simulink model "CCMLoad"  (R2022b) (continued)
== system CCMLoad/CCMLoad_Runnable_sys == (continued)
  HvacCfgForClimaCtrl_HvacCfgForHvacReBlowerType_read : Inport  Port=50
  HvacCfgForClimaCtrl_HvacCfgForHvacReVentPstn_read : Inport  Port=51
  HvacCfgForClimaCtrl_HvacCfgForHvacSensorExistSts_read : Inport  Port=52
  HvacCfgForClimaCtrl_HvacCfgForHvacSnsrRCmptmtESts_read : Inport  Port=53
  HvacCfgForClimaCtrl_HvacCfgForHvacSnsrReHexESts_read : Inport  Port=54
  HvacFanDutyReqRe_HvacFanDutyReqRe_read : Inport  Port=55
  HvacFanReqFrnt_HvacFanReqFrnt_read : Inport  Port=56
  HvacFanSts2HvacFanMotU2_HvacFanSts2HvacFanMotU2_read : Inport  Port=57
  HvacFanVoltReqFrnt_HvacFanVoltReqFrnt_read : Inport  Port=58
  HvacFanVoltReqRe_HvacFanVoltReqRe_read : Inport  Port=59
  IDX_RETXVDiagStatus_read : Inport  Port=60
  ReEvapVlvReq_ReEvapVlvReq_read : Inport  Port=61
  ReVbmrSts_ReVbmrSts_read : Inport  Port=62
  RlyCmdCmft_RlyCmdCmft_read : Inport  Port=63
  RlyCmftForClimaReq_Ngcc_RlyCmftForClimaReq_read : Inport  Port=64
  RlyCrashForClimaReq_Ngcc_RlyCrashForClimaReq_read : Inport  Port=65
  SysServCarCfgSts_SysServCCPBGMNum_read : Inport  Port=66
  SysServCarCfgSts_SysServCCPLin2AQSSts_read : Inport  Port=67
  SysServCarCfgSts_SysServDUBlowerPwmSignTypeSts_read : Inport  Port=68
  SysServCarCfgSts_SysServDUDCFLMdeSts_read : Inport  Port=69
  SysServCarCfgSts_SysServDULin4HFAFStsH_read : Inport  Port=70
  SysServCarCfgSts_SysServDUReBlowerPwmSignSts_read : Inport  Port=71
  SysServCarCfgSts_SysServDUReBlowerPwmSts_read : Inport  Port=72
  SysServCarCfgSts_SysServFrntHEXSnsrPosn_read : Inport  Port=73
  SysServCarCfgSts_SysServLowSideRETXVSts_read : Inport  Port=74
  SysServCarCfgSts_SysServReHEXSnsrPosn_read : Inport  Port=75
  SysServCarCfgSts_SysServRHFASts_read : Inport  Port=76
  SysServCarCfgSts_SysServSnsrForFCmptmtTType_read : Inport  Port=77
  TiAndDateIndcn_TiAndDateIndcn_read : Inport  Port=78
  BswLifeCycle_LifeCycle_read : Inport  Port=79
  function : TriggerPort  TriggerType=function-call  VariantControl=(inherit)  SampleTimeType=periodic  SampleTime=-1
  AdServ_AdSampleValue : FunctionCaller  FunctionPrototype=[AdValue,ERR] = AdServ_AdSampleValue()  OutputArgumentSpecifications=P_SampleValueType, P_Std_ReturnType
  AdServ_AdSampleValueSts : FunctionCaller  FunctionPrototype=[AdValue,ERR] = AdServ_AdSampleValueSts()  OutputArgumentSpecifications=P_SampleValueTypeSts, P_Std_ReturnType
  AdServ_AdcSetup : FunctionCaller  FunctionPrototype=ERR = AdServ_AdcSetup()  OutputArgumentSpecifications=P_Std_ReturnType
  DioServ_PowerOffInitChannel : FunctionCaller  FunctionPrototype=ERR = DioServ_PowerOffInitChannel()  OutputArgumentSpecifications=P_Std_ReturnType
  DioServ_PowerOnInitChannel : FunctionCaller  FunctionPrototype=ERR = DioServ_PowerOnInitChannel()  OutputArgumentSpecifications=P_Std_ReturnType
  DioServ_ReadChannel : FunctionCaller  FunctionPrototype=[Level,ERR] = DioServ_ReadChannel(ChannelId)  InputArgumentSpecifications=DioChannelType(0)  OutputArgumentSpecifications=P_DioLevelType, P_Std_ReturnType
  DioServ_WriteChannel : FunctionCaller  FunctionPrototype=ERR = DioServ_WriteChannel(ChannelId,Level)  InputArgumentSpecifications=DioChannelType(0), P_DioLevelType  OutputArgumentSpecifications=P_Std_ReturnType
  Ground_{1..24} (24 alike) : Ground
  IcuServ_GetDutyCycleValues : FunctionCaller  FunctionPrototype=[DutyCycleValues,ERR] = IcuServ_GetDutyCycleValues(Channel)  InputArgumentSpecifications=IcuChannelType(0)  OutputArgumentSpecifications=P_IcuDutyCycleType, P_Std_ReturnType
  IcuServ_IcuStart : FunctionCaller  FunctionPrototype=ERR = IcuServ_IcuStart()  OutputArgumentSpecifications=P_Std_ReturnType
  IcuServ_SetMaxPwmPeriod : FunctionCaller  FunctionPrototype=ERR = IcuServ_SetMaxPwmPeriod(Channel,MaxPwmPeriod)  InputArgumentSpecifications=IcuChannelType(0), uint16(1)  OutputArgumentSpecifications=P_Std_ReturnType
  NvMServ_HfaMtrCalReadBlock : FunctionCaller  FunctionPrototype=[Data,ERR] = NvMServ_HfaMtrCalReadBlock()  OutputArgumentSpecifications=uint8([1]), P_Std_ReturnType
  NvMServ_HfaMtrCalWriteBlock : FunctionCaller  FunctionPrototype=ERR = NvMServ_HfaMtrCalWriteBlock(Data)  InputArgumentSpecifications=uint8([1])  OutputArgumentSpecifications=P_Std_ReturnType
  NvMServ_NvMNotifyJobWorking : FunctionCaller  FunctionPrototype=[Status,ERR] = NvMServ_NvMNotifyJobWorking(BlockId)  InputArgumentSpecifications=NvMBlockId(0)  OutputArgumentSpecifications=uint8(1), P_Std_ReturnType
  NvMServ_VgaMtrCalReadBlock : FunctionCaller  FunctionPrototype=[Data,ERR] = NvMServ_VgaMtrCalReadBlock()  OutputArgumentSpecifications=uint8([1]), P_Std_ReturnType
  NvMServ_VgaMtrCalWriteBlock : FunctionCaller  FunctionPrototype=ERR = NvMServ_VgaMtrCalWriteBlock(Data)  InputArgumentSpecifications=uint8([1])  OutputArgumentSpecifications=P_Std_ReturnType
  NvMServ_WriteBlock : FunctionCaller  FunctionPrototype=ERR = NvMServ_WriteBlock(BlockId,Data)  InputArgumentSpecifications=NvMBlockId(0), uint8([1])  OutputArgumentSpecifications=P_Std_ReturnType
  PA_NvMBlockCarCfgBulkDataInit : Constant  Value=NvMBlockCarCfgBulkDataInit
  PwmServ_SetDutyCycle : FunctionCaller  FunctionPrototype=ERR = PwmServ_SetDutyCycle(ChannelNumber,u16DutyCycle)  InputArgumentSpecifications=PwmChannelType(0), P_u16DutyCycle  OutputArgumentSpecifications=P_Std_ReturnType
  Terminator_{1..104} (104 alike) : Terminator
  DutyCmdForMosBlw_DutyCmdForMosBlw_write : Outport
      settings: EnsureOutportIsVirtual=on
  DutyCmdForPWMBlw_DutyCmdForPWMBlw_write : Outport  Port=2
      settings: EnsureOutportIsVirtual=on
  HvacLpmUFbRe_HvacLpmUFbRe_write : Outport  Port=3
      settings: EnsureOutportIsVirtual=on
  HvacPwm2MosVFbFrnt_HvacPwm2MosVFbFrnt_write : Outport  Port=4
      settings: EnsureOutportIsVirtual=on
  HvacPwmNFbFrnt_HvacPwmNFbFrnt_write : Outport  Port=5
      settings: EnsureOutportIsVirtual=on
  HvacPwmSignErr_HvacPwmSignErr_write : Outport  Port=6
      settings: EnsureOutportIsVirtual=on
  NvMBlockCCMLoadDataArray_NvMBlockCCMLoadDataArray_write : Outport  Port=7
      settings: EnsureOutportIsVirtual=on
  ReEvapVlvSts_ReEvapVlvSts_write : Outport  Port=8
      settings: EnsureOutportIsVirtual=on
  ReHvacBlowerSts_ReHvacBlowerSts_write : Outport  Port=9
      settings: EnsureOutportIsVirtual=on
  AqsPwrSplySta_AqsPwrSplySta_write : Outport  Port=10
      settings: EnsureOutportIsVirtual=on
  FrntHvacBlowerSts_FrntHvacBlowerSts_write : Outport  Port=11
      settings: EnsureOutportIsVirtual=on
  wires:
  FanForCmptmtTRunReqFromClimaCtrl_FanForCmptmtTRunReqFromClimaCtrl_read/1 -> Terminator_1/1
  FrntHvacBlowerSts_HBMF_FrntHvacBlowerSts_read/1 -> Terminator_2/1
  HvacFanDutyReqFrnt_HvacFanDutyReqFrnt_read/1 -> Terminator_3/1
  LdAdVal_CurrAdVal_read/1 -> Terminator_4/1
  LdAdVal_ValidAdVal_read/1 -> Terminator_5/1
  PwrSplyToSnsrForAirQly_PwrSplyToSnsrForAirQly_read/1 -> Terminator_6/1
  SysServCarCfgSts_SysServCarID_read/1 -> Terminator_7/1
  SysServCarCfgSts_SysServCCPAQSSts_read/1 -> Terminator_8/1
  SysServCarCfgSts_SysServCCPHEXSensorSts_read/1 -> Terminator_9/1
  SysServCarCfgSts_SysServCCPLin2AFUSts_read/1 -> Terminator_10/1
  SysServCarCfgSts_SysServCCPLin2AIUSts_read/1 -> Terminator_11/1
  SysServCarCfgSts_SysServCCPLin2CDSSts_read/1 -> Terminator_12/1
  SysServCarCfgSts_SysServCCPLin2PMSISts_read/1 -> Terminator_13/1
  SysServCarCfgSts_SysServCCPLin3VGASts_read/1 -> Terminator_14/1
  SysServCarCfgSts_SysServDUAirVentSnrSts_read/1 -> Terminator_15/1
  SysServCarCfgSts_SysServDUBlowerPwmSignSts_read/1 -> Terminator_16/1
  SysServCarCfgSts_SysServDUBlowerPwmSts_read/1 -> Terminator_17/1
  SysServCarCfgSts_SysServDUDCDefrostSts_read/1 -> Terminator_18/1
  SysServCarCfgSts_SysServDUDCModeSts_read/1 -> Terminator_19/1
  SysServCarCfgSts_SysServDUDCRecSts_read/1 -> Terminator_20/1
  SysServCarCfgSts_SysServDUDCSModeSts_read/1 -> Terminator_21/1
  SysServCarCfgSts_SysServDUDCSTLSts_read/1 -> Terminator_22/1
  SysServCarCfgSts_SysServDUDCTLSts_read/1 -> Terminator_23/1
  SysServCarCfgSts_SysServDUDCTRSts_read/1 -> Terminator_24/1
  SysServCarCfgSts_SysServDUFLFootSts_read/1 -> Terminator_25/1
  SysServCarCfgSts_SysServDUFLVentSts_read/1 -> Terminator_26/1
  SysServCarCfgSts_SysServDUFRFootSts_read/1 -> Terminator_27/1
  SysServCarCfgSts_SysServDUFRVentSts_read/1 -> Terminator_28/1
  SysServCarCfgSts_SysServDUIncarMotorSts_read/1 -> Terminator_29/1
  SysServCarCfgSts_SysServDULin1HBMFSts_read/1 -> Terminator_30/1
  SysServCarCfgSts_SysServDULin4HFAFSts_read/1 -> Terminator_31/1
  SysServCarCfgSts_SysServDUSFLFootSts_read/1 -> Terminator_32/1
  SysServCarCfgSts_SysServDUSFLVentSts_read/1 -> Terminator_33/1
  SysServCarCfgSts_SysServDUSFRFootSts_read/1 -> Terminator_34/1
  SysServCarCfgSts_SysServDUSFRVentSts_read/1 -> Terminator_35/1
  SysServCarCfgSts_SysServDUSnsrForFCmptmtTSts_read/1 -> Terminator_36/1
  SysServCarCfgSts_SysServDUSnsrForRair_read/1 -> Terminator_37/1
  SysServCarCfgSts_SysServDUSnsrForRCmptmtTSts_read/1 -> Terminator_38/1
  VehModMngtGlbSafe1_VehModMngtGlbSafe1_read/1 -> Terminator_39/1
  BatLoadBtrMSts_BatLoadBtrMSts_read/1 -> Terminator_40/1
  BatSysBtrMSts_BatSysBtrMSts_read/1 -> Terminator_41/1
  BattAdVal_CurrAdVal_read/1 -> Terminator_42/1
  BattAdVal_ValidAdVal_read/1 -> Terminator_43/1
  ClimRlyCmd_ClimRlyCmd_read/1 -> Terminator_44/1
  HvacCfgForClimaCtrl_HvacCfgForAQSTypes_read/1 -> Terminator_45/1
  HvacCfgForClimaCtrl_HvacCfgForHvacFrontBlowerType_read/1 -> Terminator_46/1
  HvacCfgForClimaCtrl_HvacCfgForHvacLowSideRETXVESts_read/1 -> Terminator_47/1
  HvacCfgForClimaCtrl_HvacCfgForHvacMiscExistSts_read/1 -> Terminator_48/1
  HvacCfgForClimaCtrl_HvacCfgForHvacMotorExistSts_read/1 -> Terminator_49/1
  HvacCfgForClimaCtrl_HvacCfgForHvacReBlowerType_read/1 -> Terminator_50/1
  HvacCfgForClimaCtrl_HvacCfgForHvacReVentPstn_read/1 -> Terminator_51/1
  HvacCfgForClimaCtrl_HvacCfgForHvacSensorExistSts_read/1 -> Terminator_52/1
  HvacCfgForClimaCtrl_HvacCfgForHvacSnsrRCmptmtESts_read/1 -> Terminator_53/1
  HvacCfgForClimaCtrl_HvacCfgForHvacSnsrReHexESts_read/1 -> Terminator_54/1
  HvacFanDutyReqRe_HvacFanDutyReqRe_read/1 -> Terminator_55/1
  HvacFanReqFrnt_HvacFanReqFrnt_read/1 -> Terminator_56/1
  HvacFanSts2HvacFanMotU2_HvacFanSts2HvacFanMotU2_read/1 -> Terminator_57/1
  HvacFanVoltReqFrnt_HvacFanVoltReqFrnt_read/1 -> Terminator_58/1
  HvacFanVoltReqRe_HvacFanVoltReqRe_read/1 -> Terminator_59/1
  IDX_RETXVDiagStatus_read/1 -> Terminator_60/1
  ReEvapVlvReq_ReEvapVlvReq_read/1 -> Terminator_61/1
  ReVbmrSts_ReVbmrSts_read/1 -> Terminator_62/1
  RlyCmdCmft_RlyCmdCmft_read/1 -> Terminator_63/1
  RlyCmftForClimaReq_Ngcc_RlyCmftForClimaReq_read/1 -> Terminator_64/1
  RlyCrashForClimaReq_Ngcc_RlyCrashForClimaReq_read/1 -> Terminator_65/1
  SysServCarCfgSts_SysServCCPBGMNum_read/1 -> Terminator_66/1
  SysServCarCfgSts_SysServCCPLin2AQSSts_read/1 -> Terminator_67/1
  SysServCarCfgSts_SysServDUBlowerPwmSignTypeSts_read/1 -> Terminator_68/1
  SysServCarCfgSts_SysServDUDCFLMdeSts_read/1 -> Terminator_69/1
  SysServCarCfgSts_SysServDULin4HFAFStsH_read/1 -> Terminator_70/1
  SysServCarCfgSts_SysServDUReBlowerPwmSignSts_read/1 -> Terminator_71/1
  SysServCarCfgSts_SysServDUReBlowerPwmSts_read/1 -> Terminator_72/1
  SysServCarCfgSts_SysServFrntHEXSnsrPosn_read/1 -> Terminator_73/1
  SysServCarCfgSts_SysServLowSideRETXVSts_read/1 -> Terminator_74/1
  SysServCarCfgSts_SysServReHEXSnsrPosn_read/1 -> Terminator_75/1
  SysServCarCfgSts_SysServRHFASts_read/1 -> Terminator_76/1
  SysServCarCfgSts_SysServSnsrForFCmptmtTType_read/1 -> Terminator_77/1
  TiAndDateIndcn_TiAndDateIndcn_read/1 -> Terminator_78/1
  BswLifeCycle_LifeCycle_read/1 -> Terminator_79/1
  AdServ_AdSampleValue/1 -> Terminator_80/1
  AdServ_AdSampleValue/2 -> Terminator_81/1
  AdServ_AdSampleValueSts/1 -> Terminator_82/1
  AdServ_AdSampleValueSts/2 -> Terminator_83/1
  AdServ_AdcSetup/1 -> Terminator_84/1
  DioServ_PowerOffInitChannel/1 -> Terminator_85/1
  DioServ_PowerOnInitChannel/1 -> Terminator_86/1
  Ground_1/1 -> DioServ_ReadChannel/1
  DioServ_ReadChannel/1 -> Terminator_87/1
  DioServ_ReadChannel/2 -> Terminator_88/1
  Ground_2/1 -> DioServ_WriteChannel/1
  Ground_3/1 -> DioServ_WriteChannel/2
  DioServ_WriteChannel/1 -> Terminator_89/1
  Ground_4/1 -> IcuServ_GetDutyCycleValues/1
  IcuServ_GetDutyCycleValues/1 -> Terminator_90/1
  IcuServ_GetDutyCycleValues/2 -> Terminator_91/1
  IcuServ_IcuStart/1 -> Terminator_92/1
  Ground_5/1 -> IcuServ_SetMaxPwmPeriod/1
  Ground_6/1 -> IcuServ_SetMaxPwmPeriod/2
  IcuServ_SetMaxPwmPeriod/1 -> Terminator_93/1
  NvMServ_HfaMtrCalReadBlock/1 -> Terminator_94/1
  NvMServ_HfaMtrCalReadBlock/2 -> Terminator_95/1
  Ground_7/1 -> NvMServ_HfaMtrCalWriteBlock/1
  NvMServ_HfaMtrCalWriteBlock/1 -> Terminator_96/1
  Ground_8/1 -> NvMServ_NvMNotifyJobWorking/1
  NvMServ_NvMNotifyJobWorking/1 -> Terminator_97/1
  NvMServ_NvMNotifyJobWorking/2 -> Terminator_98/1
  NvMServ_VgaMtrCalReadBlock/1 -> Terminator_99/1
  NvMServ_VgaMtrCalReadBlock/2 -> Terminator_100/1
  Ground_9/1 -> NvMServ_VgaMtrCalWriteBlock/1
  NvMServ_VgaMtrCalWriteBlock/1 -> Terminator_101/1
  Ground_10/1 -> NvMServ_WriteBlock/1
  Ground_11/1 -> NvMServ_WriteBlock/2
  NvMServ_WriteBlock/1 -> Terminator_102/1
  PA_NvMBlockCarCfgBulkDataInit/1 -> Terminator_103/1
  Ground_12/1 -> PwmServ_SetDutyCycle/1
  Ground_13/1 -> PwmServ_SetDutyCycle/2
  PwmServ_SetDutyCycle/1 -> Terminator_104/1
  Ground_14/1 -> DutyCmdForMosBlw_DutyCmdForMosBlw_write/1
  Ground_15/1 -> DutyCmdForPWMBlw_DutyCmdForPWMBlw_write/1
  Ground_16/1 -> HvacLpmUFbRe_HvacLpmUFbRe_write/1
  Ground_17/1 -> HvacPwm2MosVFbFrnt_HvacPwm2MosVFbFrnt_write/1
  Ground_18/1 -> HvacPwmNFbFrnt_HvacPwmNFbFrnt_write/1
  Ground_19/1 -> HvacPwmSignErr_HvacPwmSignErr_write/1
  Ground_20/1 -> NvMBlockCCMLoadDataArray_NvMBlockCCMLoadDataArray_write/1
  Ground_21/1 -> ReEvapVlvSts_ReEvapVlvSts_write/1
  Ground_22/1 -> ReHvacBlowerSts_ReHvacBlowerSts_write/1
  Ground_23/1 -> AqsPwrSplySta_AqsPwrSplySta_write/1
  Ground_24/1 -> FrntHvacBlowerSts_FrntHvacBlowerSts_write/1

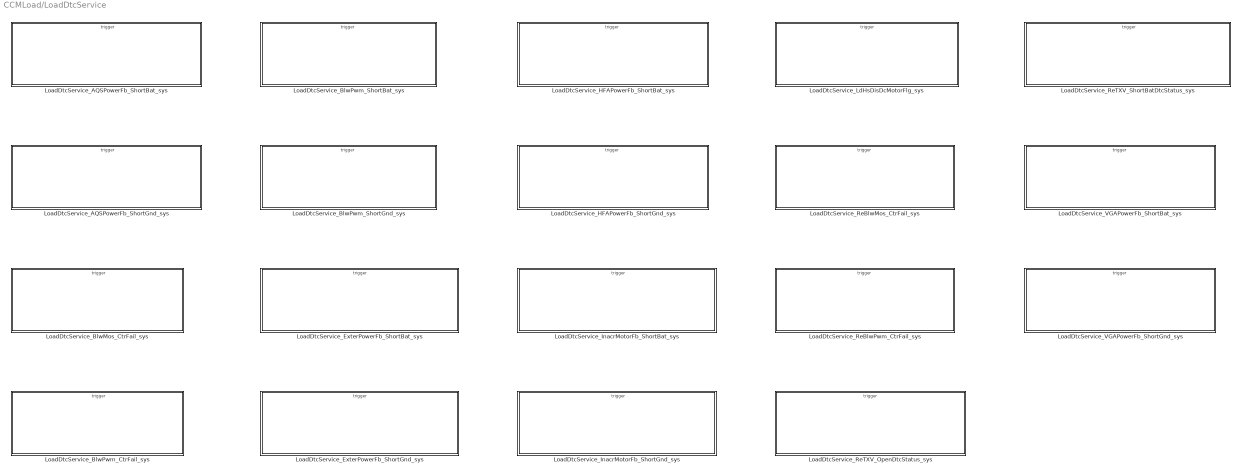
== system CCMLoad/LoadDtcService ==
  LoadDtcService_AQSPowerFb_ShortBat_sys : Subsystem
  LoadDtcService_AQSPowerFb_ShortGnd_sys : Subsystem
  LoadDtcService_BlwMos_CtrFail_sys : Subsystem
  LoadDtcService_BlwPwm_CtrFail_sys : Subsystem
  LoadDtcService_BlwPwm_ShortBat_sys : Subsystem
  LoadDtcService_BlwPwm_ShortGnd_sys : Subsystem
  LoadDtcService_ExterPowerFb_ShortBat_sys : Subsystem
  LoadDtcService_ExterPowerFb_ShortGnd_sys : Subsystem
  LoadDtcService_HFAPowerFb_ShortBat_sys : Subsystem
  LoadDtcService_HFAPowerFb_ShortGnd_sys : Subsystem
  LoadDtcService_InacrMotorFb_ShortBat_sys : Subsystem
  LoadDtcService_InacrMotorFb_ShortGnd_sys : Subsystem
  LoadDtcService_LdHsDisDcMotorFlg_sys : Subsystem
  LoadDtcService_ReBlwMos_CtrFail_sys : Subsystem
  LoadDtcService_ReBlwPwm_CtrFail_sys : Subsystem
  LoadDtcService_ReTXV_OpenDtcStatus_sys : Subsystem
  LoadDtcService_ReTXV_ShortBatDtcStatus_sys : Subsystem
  LoadDtcService_VGAPowerFb_ShortBat_sys : Subsystem
  LoadDtcService_VGAPowerFb_ShortGnd_sys : Subsystem

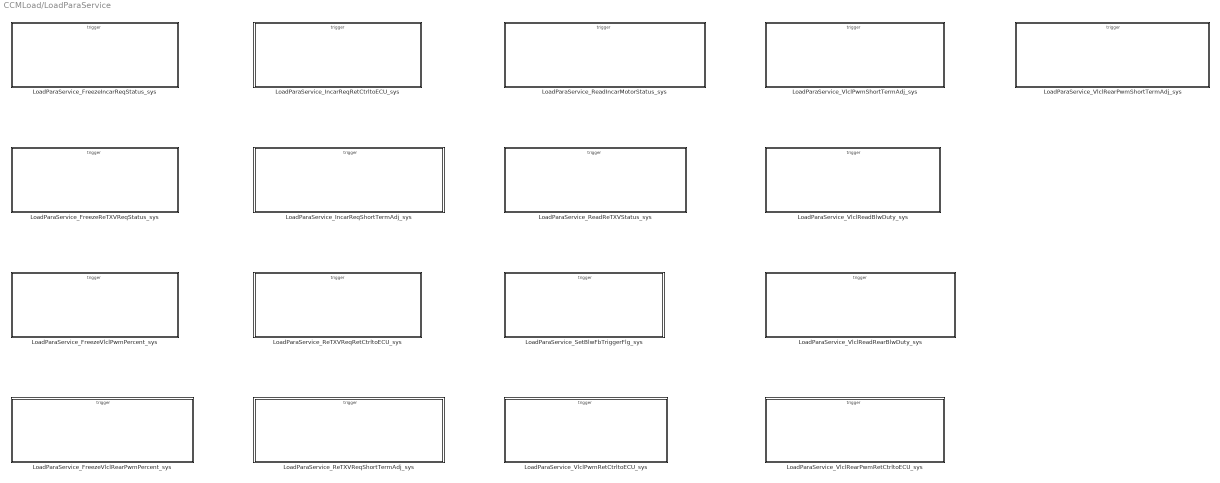
== system CCMLoad/LoadParaService ==
  LoadParaService_FreezeIncarReqStatus_sys : Subsystem
  LoadParaService_FreezeReTXVReqStatus_sys : Subsystem
  LoadParaService_FreezeVlclPwmPercent_sys : Subsystem
  LoadParaService_FreezeVlclRearPwmPercent_sys : Subsystem
  LoadParaService_IncarReqRetCtrltoECU_sys : Subsystem
  LoadParaService_IncarReqShortTermAdj_sys : Subsystem
  LoadParaService_ReTXVReqRetCtrltoECU_sys : Subsystem
  LoadParaService_ReTXVReqShortTermAdj_sys : Subsystem
  LoadParaService_ReadIncarMotorStatus_sys : Subsystem
  LoadParaService_ReadReTXVStatus_sys : Subsystem
  LoadParaService_SetBlwFbTriggerFlg_sys : Subsystem
  LoadParaService_VlclPwmRetCtrltoECU_sys : Subsystem
  LoadParaService_VlclPwmShortTermAdj_sys : Subsystem
  LoadParaService_VlclReadBlwDuty_sys : Subsystem
  LoadParaService_VlclReadRearBlwDuty_sys : Subsystem
  LoadParaService_VlclRearPwmRetCtrltoECU_sys : Subsystem
  LoadParaService_VlclRearPwmShortTermAdj_sys : Subsystem

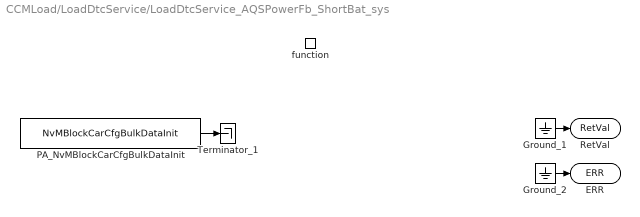
== system CCMLoad/LoadDtcService/LoadDtcService_AQSPowerFb_ShortBat_sys ==
  function : TriggerPort  TriggerType=function-call  IsSimulinkFunction=on  FunctionName=LoadDtcService_AQSPowerFb_ShortBat  VariantControl=(inherit)
  Ground_1 : Ground
  Ground_2 : Ground
  PA_NvMBlockCarCfgBulkDataInit : Constant  Value=NvMBlockCarCfgBulkDataInit
  Terminator_1 : Terminator
  RetVal : ArgOut  OutDataTypeStr=Enum: DTCState  ArgumentName=RetVal
  ERR : ArgOut  Port=2  OutDataTypeStr=Std_ReturnType  ArgumentName=ERR
  wires:
  PA_NvMBlockCarCfgBulkDataInit/1 -> Terminator_1/1
  Ground_1/1 -> RetVal/1
  Ground_2/1 -> ERR/1

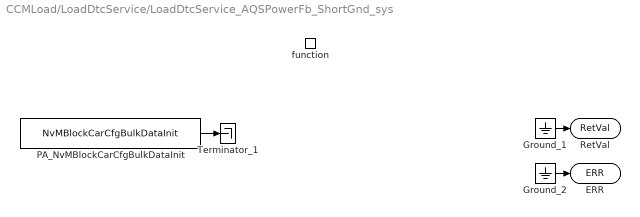
== system CCMLoad/LoadDtcService/LoadDtcService_AQSPowerFb_ShortGnd_sys ==
  function : TriggerPort  TriggerType=function-call  IsSimulinkFunction=on  FunctionName=LoadDtcService_AQSPowerFb_ShortGnd  VariantControl=(inherit)
  Ground_1 : Ground
  Ground_2 : Ground
  PA_NvMBlockCarCfgBulkDataInit : Constant  Value=NvMBlockCarCfgBulkDataInit
  Terminator_1 : Terminator
  RetVal : ArgOut  OutDataTypeStr=Enum: DTCState  ArgumentName=RetVal
  ERR : ArgOut  Port=2  OutDataTypeStr=Std_ReturnType  ArgumentName=ERR
  wires:
  PA_NvMBlockCarCfgBulkDataInit/1 -> Terminator_1/1
  Ground_1/1 -> RetVal/1
  Ground_2/1 -> ERR/1

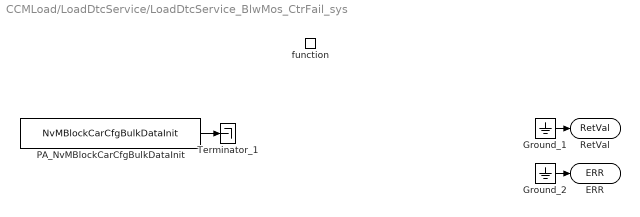
== system CCMLoad/LoadDtcService/LoadDtcService_BlwMos_CtrFail_sys ==
  function : TriggerPort  TriggerType=function-call  IsSimulinkFunction=on  FunctionName=LoadDtcService_BlwMos_CtrFail  VariantControl=(inherit)
  Ground_1 : Ground
  Ground_2 : Ground
  PA_NvMBlockCarCfgBulkDataInit : Constant  Value=NvMBlockCarCfgBulkDataInit
  Terminator_1 : Terminator
  RetVal : ArgOut  OutDataTypeStr=Enum: DTCState  ArgumentName=RetVal
  ERR : ArgOut  Port=2  OutDataTypeStr=Std_ReturnType  ArgumentName=ERR
  wires:
  PA_NvMBlockCarCfgBulkDataInit/1 -> Terminator_1/1
  Ground_1/1 -> RetVal/1
  Ground_2/1 -> ERR/1

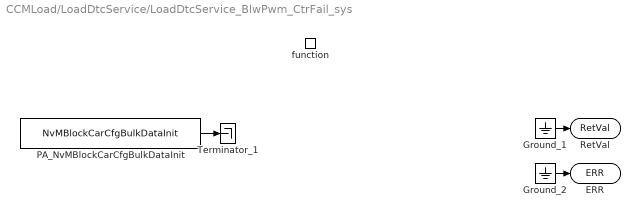
== system CCMLoad/LoadDtcService/LoadDtcService_BlwPwm_CtrFail_sys ==
  function : TriggerPort  TriggerType=function-call  IsSimulinkFunction=on  FunctionName=LoadDtcService_BlwPwm_CtrFail  VariantControl=(inherit)
  Ground_1 : Ground
  Ground_2 : Ground
  PA_NvMBlockCarCfgBulkDataInit : Constant  Value=NvMBlockCarCfgBulkDataInit
  Terminator_1 : Terminator
  RetVal : ArgOut  OutDataTypeStr=Enum: DTCState  ArgumentName=RetVal
  ERR : ArgOut  Port=2  OutDataTypeStr=Std_ReturnType  ArgumentName=ERR
  wires:
  PA_NvMBlockCarCfgBulkDataInit/1 -> Terminator_1/1
  Ground_1/1 -> RetVal/1
  Ground_2/1 -> ERR/1

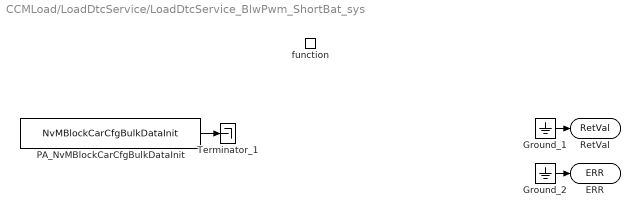
== system CCMLoad/LoadDtcService/LoadDtcService_BlwPwm_ShortBat_sys ==
  function : TriggerPort  TriggerType=function-call  IsSimulinkFunction=on  FunctionName=LoadDtcService_BlwPwm_ShortBat  VariantControl=(inherit)
  Ground_1 : Ground
  Ground_2 : Ground
  PA_NvMBlockCarCfgBulkDataInit : Constant  Value=NvMBlockCarCfgBulkDataInit
  Terminator_1 : Terminator
  RetVal : ArgOut  OutDataTypeStr=Enum: DTCState  ArgumentName=RetVal
  ERR : ArgOut  Port=2  OutDataTypeStr=Std_ReturnType  ArgumentName=ERR
  wires:
  PA_NvMBlockCarCfgBulkDataInit/1 -> Terminator_1/1
  Ground_1/1 -> RetVal/1
  Ground_2/1 -> ERR/1

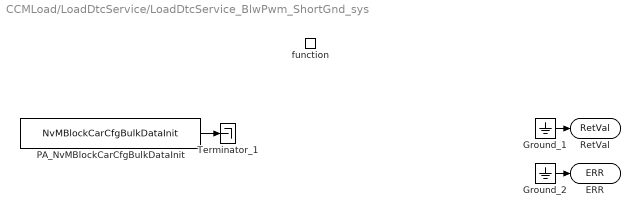
== system CCMLoad/LoadDtcService/LoadDtcService_BlwPwm_ShortGnd_sys ==
  function : TriggerPort  TriggerType=function-call  IsSimulinkFunction=on  FunctionName=LoadDtcService_BlwPwm_ShortGnd  VariantControl=(inherit)
  Ground_1 : Ground
  Ground_2 : Ground
  PA_NvMBlockCarCfgBulkDataInit : Constant  Value=NvMBlockCarCfgBulkDataInit
  Terminator_1 : Terminator
  RetVal : ArgOut  OutDataTypeStr=Enum: DTCState  ArgumentName=RetVal
  ERR : ArgOut  Port=2  OutDataTypeStr=Std_ReturnType  ArgumentName=ERR
  wires:
  PA_NvMBlockCarCfgBulkDataInit/1 -> Terminator_1/1
  Ground_1/1 -> RetVal/1
  Ground_2/1 -> ERR/1

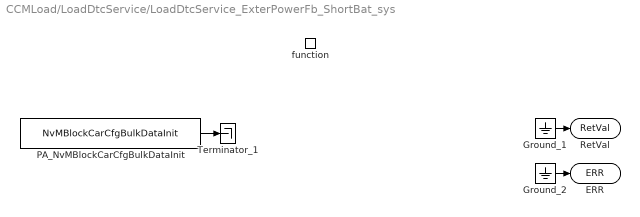
== system CCMLoad/LoadDtcService/LoadDtcService_ExterPowerFb_ShortBat_sys ==
  function : TriggerPort  TriggerType=function-call  IsSimulinkFunction=on  FunctionName=LoadDtcService_ExterPowerFb_ShortBat  VariantControl=(inherit)
  Ground_1 : Ground
  Ground_2 : Ground
  PA_NvMBlockCarCfgBulkDataInit : Constant  Value=NvMBlockCarCfgBulkDataInit
  Terminator_1 : Terminator
  RetVal : ArgOut  OutDataTypeStr=Enum: DTCState  ArgumentName=RetVal
  ERR : ArgOut  Port=2  OutDataTypeStr=Std_ReturnType  ArgumentName=ERR
  wires:
  PA_NvMBlockCarCfgBulkDataInit/1 -> Terminator_1/1
  Ground_1/1 -> RetVal/1
  Ground_2/1 -> ERR/1

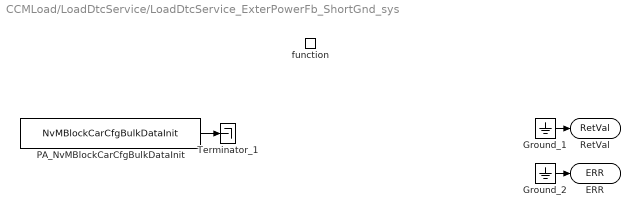
== system CCMLoad/LoadDtcService/LoadDtcService_ExterPowerFb_ShortGnd_sys ==
  function : TriggerPort  TriggerType=function-call  IsSimulinkFunction=on  FunctionName=LoadDtcService_ExterPowerFb_ShortGnd  VariantControl=(inherit)
  Ground_1 : Ground
  Ground_2 : Ground
  PA_NvMBlockCarCfgBulkDataInit : Constant  Value=NvMBlockCarCfgBulkDataInit
  Terminator_1 : Terminator
  RetVal : ArgOut  OutDataTypeStr=Enum: DTCState  ArgumentName=RetVal
  ERR : ArgOut  Port=2  OutDataTypeStr=Std_ReturnType  ArgumentName=ERR
  wires:
  PA_NvMBlockCarCfgBulkDataInit/1 -> Terminator_1/1
  Ground_1/1 -> RetVal/1
  Ground_2/1 -> ERR/1

== system CCMLoad/LoadDtcService/LoadDtcService_HFAPowerFb_ShortBat_sys ==
  function : TriggerPort  TriggerType=function-call  IsSimulinkFunction=on  FunctionName=LoadDtcService_HFAPowerFb_ShortBat  VariantControl=(inherit)
  Ground_1 : Ground
  Ground_2 : Ground
  PA_NvMBlockCarCfgBulkDataInit : Constant  Value=NvMBlockCarCfgBulkDataInit
  Terminator_1 : Terminator
  RetVal : ArgOut  OutDataTypeStr=Enum: DTCState  ArgumentName=RetVal
  ERR : ArgOut  Port=2  OutDataTypeStr=Std_ReturnType  ArgumentName=ERR
  wires:
  PA_NvMBlockCarCfgBulkDataInit/1 -> Terminator_1/1
  Ground_1/1 -> RetVal/1
  Ground_2/1 -> ERR/1

== system CCMLoad/LoadDtcService/LoadDtcService_HFAPowerFb_ShortGnd_sys ==
  function : TriggerPort  TriggerType=function-call  IsSimulinkFunction=on  FunctionName=LoadDtcService_HFAPowerFb_ShortGnd  VariantControl=(inherit)
  Ground_1 : Ground
  Ground_2 : Ground
  PA_NvMBlockCarCfgBulkDataInit : Constant  Value=NvMBlockCarCfgBulkDataInit
  Terminator_1 : Terminator
  RetVal : ArgOut  OutDataTypeStr=Enum: DTCState  ArgumentName=RetVal
  ERR : ArgOut  Port=2  OutDataTypeStr=Std_ReturnType  ArgumentName=ERR
  wires:
  PA_NvMBlockCarCfgBulkDataInit/1 -> Terminator_1/1
  Ground_1/1 -> RetVal/1
  Ground_2/1 -> ERR/1

== system CCMLoad/LoadDtcService/LoadDtcService_InacrMotorFb_ShortBat_sys ==
  function : TriggerPort  TriggerType=function-call  IsSimulinkFunction=on  FunctionName=LoadDtcService_InacrMotorFb_ShortBat  VariantControl=(inherit)
  Ground_1 : Ground
  Ground_2 : Ground
  PA_NvMBlockCarCfgBulkDataInit : Constant  Value=NvMBlockCarCfgBulkDataInit
  Terminator_1 : Terminator
  RetVal : ArgOut  OutDataTypeStr=Enum: DTCState  ArgumentName=RetVal
  ERR : ArgOut  Port=2  OutDataTypeStr=Std_ReturnType  ArgumentName=ERR
  wires:
  PA_NvMBlockCarCfgBulkDataInit/1 -> Terminator_1/1
  Ground_1/1 -> RetVal/1
  Ground_2/1 -> ERR/1

== system CCMLoad/LoadDtcService/LoadDtcService_InacrMotorFb_ShortGnd_sys ==
  function : TriggerPort  TriggerType=function-call  IsSimulinkFunction=on  FunctionName=LoadDtcService_InacrMotorFb_ShortGnd  VariantControl=(inherit)
  Ground_1 : Ground
  Ground_2 : Ground
  PA_NvMBlockCarCfgBulkDataInit : Constant  Value=NvMBlockCarCfgBulkDataInit
  Terminator_1 : Terminator
  RetVal : ArgOut  OutDataTypeStr=Enum: DTCState  ArgumentName=RetVal
  ERR : ArgOut  Port=2  OutDataTypeStr=Std_ReturnType  ArgumentName=ERR
  wires:
  PA_NvMBlockCarCfgBulkDataInit/1 -> Terminator_1/1
  Ground_1/1 -> RetVal/1
  Ground_2/1 -> ERR/1

== system CCMLoad/LoadDtcService/LoadDtcService_LdHsDisDcMotorFlg_sys ==
  function : TriggerPort  TriggerType=function-call  IsSimulinkFunction=on  FunctionName=LoadDtcService_LdHsDisDcMotorFlg  VariantControl=(inherit)
  Ground_1 : Ground
  Ground_2 : Ground
  PA_NvMBlockCarCfgBulkDataInit : Constant  Value=NvMBlockCarCfgBulkDataInit
  Terminator_1 : Terminator
  Status : ArgOut  OutDataTypeStr=Enum: DTCState  ArgumentName=Status
  ERR : ArgOut  Port=2  OutDataTypeStr=Std_ReturnType  ArgumentName=ERR
  wires:
  PA_NvMBlockCarCfgBulkDataInit/1 -> Terminator_1/1
  Ground_1/1 -> Status/1
  Ground_2/1 -> ERR/1
